simulink model "ofdm_txrx_mimo"  (Simulink 7.1)
settings: Solver=ode45  StartTime=0.0  StopTime=6e3  RelTol=1e-3
PreLoadFcn:
  | ofdm_rx_mimo_init
InitFcn:
  | ofdm_rx_mimo_init
StartFcn:
  | ofdm_rx_mimo_init
values from neighbouring files (symbols this model uses):
  BER_RAM_init_size = 2048    [ofdm_tx_mimo_init.m]
  CPLength = 16    [ofdm_tx_mimo_init.m]
  CRCPolynomial = hex2dec('04c11db7')    [ofdm_tx_mimo_init.m]
  freqOffset_track_filtCoef_i = hex2dec('2500')/2^32    [ofdm_rx_mimo_init.m]
  freqOffset_track_filtCoef_p = hex2dec('d0000')/2^32    [ofdm_rx_mimo_init.m]
  hi = zeros(1,length(cci))    [ofdm_rx_mimo_init.m]
  hr = zeros(1,length(ccr))    [ofdm_rx_mimo_init.m]
  max_CP_length = 16    [ofdm_tx_mimo_init.m]
  max_num_baseRateSymbols = 31    [ofdm_tx_mimo_init.m]
  max_num_subcarriers = 64    [ofdm_tx_mimo_init.m]
  max_num_trainingSymbols = 15    [ofdm_tx_mimo_init.m]
  max_numBytes = 16384    [ofdm_tx_mimo_init.m]
  max_OFDM_symbols = 2047    [ofdm_tx_mimo_init.m]
  modConstellation_qam16 = .75*[1 3 -1 -3]./3    [ofdm_tx_mimo_init.m]
  modConstellation_qam256 = 0.9375*[3 1 5 7 11 9 13 15 -3 -1 -5 -7 -11 -9 -13 -15]./15    [ofdm_tx_mimo_init.m]
  modConstellation_qam64 = 0.875*[3 1 5 7 -3 -1 -5 -7]./7    [ofdm_tx_mimo_init.m]
  modConstellation_qpsk = [1 -1]./sqrt(2)    [ofdm_tx_mimo_init.m]
  numBaseRateSymbols = 2    [ofdm_tx_mimo_init.m]
  numSubcarriers = 64    [ofdm_tx_mimo_init.m]
  numTrainingSymbols = 2    [ofdm_tx_mimo_init.m]
  pilot1_indicies = 7 + ( (64-7) * 2^16)    (= 3735559)    [ofdm_tx_mimo_init.m]
  pilot2_indicies = 21 + ( (64-21) * 2^16)    (= 2818069)    [ofdm_tx_mimo_init.m]
  pilotValue_neg = hex2dec('8000') + (2^16 * 0)    [ofdm_tx_mimo_init.m]
  pilotValue_pos = hex2dec('7FFF') + (2^16 * 0)    [ofdm_tx_mimo_init.m]
  pkt_crossCorr_thresh = 0.7    [ofdm_rx_mimo_init.m]
  pkt_energy_thresh = 0    [ofdm_rx_mimo_init.m]
  pkt_numPayloadBytes = 1400+42-6    (= 1436)    [ofdm_tx_mimo_init.m]
  pktByteNums = numHeaderBytes +  				byteIndex_numPayloadBytes(1)*2^8 +  				byteIndex_numPayloadBytes(2)*2^16 +  				byteIndex_simpleDynModMasks * 2^24    [ofdm_rx_mimo_init.m]
  PktDet_Delay = 26 + Symbol_Timing_Offset*2^7    (= 1050)    [ofdm_rx_mimo_init.m]
  preamble = 6*[0 shortsyms_10 longsyms_2]    [ofdm_tx_mimo_init.m]
  preamble_I = real(preamble)    [ofdm_tx_mimo_init.m]
  preamble_Q = imag(preamble)    [ofdm_tx_mimo_init.m]
  RAM_init_size = max_numBytes    (= 16384)    [ofdm_tx_mimo_init.m]
  reg_Interrupt_PktBuf_Control = reg_InterruptControl +  	1 * 2^16 +  	0 * 2^24    (= 65656)    [ofdm_rx_mimo_init.m]
  rx_controlBits = 1 * 2  		 + 1 * 4  		 + 0 * 8  		 + 1 * 16 * rx_SISO_Mode  		 + 1 * 32  		 + 1 * 64  		 + 0 * 128  		 + 1 * 256  		 + 0 * 512  		 + 1 * 1024  		 + 0 * 2048  		 + 1 * 4096  		 + 1 * 8192  		 + 0 * 16384  		 + 1 * 65536  		 + 1 * 131072  		 + 0 * 262144  		 + 1 * 524288    [ofdm_rx_mimo_init.m]
  Rx_PhaseNoiseTrack_K = 0.6094    [ofdm_rx_mimo_init.m]
  rx_postEq_scaling = round(rxScaling*2^11) * (1 + 2^16)    [ofdm_rx_mimo_init.m]
  RxDataScrambling_Seq = [40 198 78 63 82 173 102 245 48 111 172 115 147 230 216 93 72 65 62 2 205 242 122 90 128 83 105 97 73 10 5 252]    [ofdm_tx_mimo_init.m]
  simOnly_numSamples = length(preamble)+( (numSubcarriers+CPLength)*(numTrainingSymbols + numBaseRateSymbols + numFullRateSymbols/2) )    [ofdm_tx_mimo_init.m]
  train = [train train]    [ofdm_tx_mimo_init.m]
  tx_controlBits = (antB_preambleShift * 2^4) + (2^0 * tx_SISO_Mode) + 4*0 + 8*1    (= 73)    [ofdm_tx_mimo_init.m]
  TxDataScrambling_Seq = [40 198 78 63 82 173 102 245 48 111 172 115 147 230 216 93 72 65 62 2 205 242 122 90 128 83 105 97 73 10 5 252]    [ofdm_tx_mimo_init.m]
  txReg_symbolCounts = (2^16 * numFullRateSymbols) + (2^8 *numBaseRateSymbols ) + numTrainingSymbols    [ofdm_tx_mimo_init.m]
  TxRx_FFTScaling = bin2dec('010111') + (bin2dec('000101') * 2^6)    [ofdm_tx_mimo_init.m]
undefined symbols (not defined in the file or in its folder): AntA, AntB, BaseRate, CRC_table_gen, gcbh, intfilt, pktTiming_count_payloadStart, pktTiming_symbol_length, xlBlockLoadCallback, xlGetSimulinkPeriod
libraries: xbsIndex_r4 x4212, dspmlti4 x4, dspsigops x4, commchan3 x2, simulink x2, commrflib2 x2, dspsrcs4 x1
(part 5 of 43)

== system ofdm_txrx_mimo/OFDM Rx MIMO/Long Corr 4x ==
  note: changed latency to zero
  note: changed latency to 0
  I In : Inport  OutDataType=sfix(16)  OutScaling=2^0
  Q In : Inport  Port=2  OutDataType=sfix(16)  OutScaling=2^0
  AddSub : xbsIndex_r4/AddSub  mode=Subtraction  use_carryin=off  use_carryout=off  en=off  latency=0  precision=Full  arith_type=Unsigned  n_bits=8  bin_pt=2  quantization=Truncate  overflow=Wrap  dbl_ovrd=off  use_behavioral_HDL=on  pipelined=off  use_rpm=off  hw_selection=Fabric  block_type=addsub
      settings: xl_area=[5 0 0 8 0 0 0]  block_version=VER_STRING_GOES_HERE
  AddSub1 : xbsIndex_r4/AddSub  mode=Addition  use_carryin=off  use_carryout=off  en=off  latency=0  precision=Full  arith_type=Unsigned  n_bits=8  bin_pt=2  quantization=Truncate  overflow=Wrap  dbl_ovrd=off  use_behavioral_HDL=on  pipelined=off  use_rpm=off  hw_selection=Fabric  block_type=addsub
      settings: xl_area=[5 0 0 8 0 0 0]  block_version=VER_STRING_GOES_HERE
  AddSub2 : xbsIndex_r4/AddSub  mode=Addition  use_carryin=off  use_carryout=off  en=off  latency=0  precision=Full  arith_type=Unsigned  n_bits=8  bin_pt=2  quantization=Truncate  overflow=Wrap  dbl_ovrd=off  use_behavioral_HDL=on  pipelined=off  use_rpm=off  hw_selection=Fabric  block_type=addsub
      settings: xl_area=[10 0 0 18 0 0 0]  block_version=VER_STRING_GOES_HERE
  C1 : Subsystem  AncestorBlock=ofdm_rx_pom36_cfglib/correlator2/Original Subsystem/C1  mask{ns=0, N=4, h=hr(1:65), Ts_In=4}
      mask prompts: ns "Num sub-correlator"; N "Coefficients/correlator"; h "Coefficients"; Ts_In "Input sample period"
  C4 : Subsystem  AncestorBlock=ofdm_rx_pom36_cfglib/correlator2/Original Subsystem/C1  mask{ns=0, N=4, h=hi(1:65), Ts_In=4}
      mask prompts: ns "Num sub-correlator"; N "Coefficients/correlator"; h "Coefficients"; Ts_In "Input sample period"
  C6 : Subsystem  AncestorBlock=ofdm_rx_pom36_cfglib/correlator2/Original Subsystem/C1  mask{ns=0, N=4, h=hi(1:65), Ts_In=4}
      mask prompts: ns "Num sub-correlator"; N "Coefficients/correlator"; h "Coefficients"; Ts_In "Input sample period"
  C7 : Subsystem  AncestorBlock=ofdm_rx_pom36_cfglib/correlator2/Original Subsystem/C1  mask{ns=0, N=4, h=hr(1:65), Ts_In=4}
      mask prompts: ns "Num sub-correlator"; N "Coefficients/correlator"; h "Coefficients"; Ts_In "Input sample period"
  Gateway Out{3..8} (6 alike) : xbsIndex_r4/Gateway Out  hdl_port=off  timing_constraint=None  locs_specified=off  LOCs={}  block_type=gatewayout
      settings: infoedit="Gateway out block.  Converts Xilinx fixed point inputs into ouputs of type Simulink integer, double, or fixed point.<P><P>Hardware notes:  In hardware these blocks become top level output ports or are discarded, depending on how they are configured."  xl_area=[0 0 0 0 0 0 0]  block_version=VER_STRING_GOES_HERE
  Long Corr Scope4x : Scope  NumInputPorts=6
      settings: YMin=-1~-1~-30~-30~-20~-25  YMax=1~1~70~70~20~20  SaveName=ScopeData2
  Mult : xbsIndex_r4/Mult  precision=Full  arith_type="Signed  (2's comp)"  n_bits=8  bin_pt=2  quantization=Truncate  overflow=Wrap  en=off  latency=1  dbl_ovrd=off  use_behavioral_HDL=off  use_embedded=on  opt=Speed  optimum_pipeline=off  pipeline=on  use_rpm=off  placement_style=Rectangular shape  block_type=mult
      settings: infoedit=Hardware notes: To use the internal pipeline stage of the dedicated multiplier you must select 'Pipeline for maximum performance'.  xl_area=[60 18 0 106 0 0 0]  block_version=VER_STRING_GOES_HERE
  Mult1 : xbsIndex_r4/Mult  precision=Full  arith_type="Signed  (2's comp)"  n_bits=8  bin_pt=2  quantization=Truncate  overflow=Wrap  en=off  latency=1  dbl_ovrd=off  use_behavioral_HDL=off  use_embedded=on  opt=Speed  optimum_pipeline=off  pipeline=on  use_rpm=off  placement_style=Rectangular shape  block_type=mult
      settings: infoedit=Hardware notes: To use the internal pipeline stage of the dedicated multiplier you must select 'Pipeline for maximum performance'.  xl_area=[60 18 0 106 0 0 0]  block_version=VER_STRING_GOES_HERE
  Threshold1 : xbsIndex_r4/Threshold  en=off  latency=0  dbl_ovrd=off  block_type=sgn
      settings: infoedit="Presents -1 as output if input is negative; otherwise presents 1.  Output is a two bit signed number."  xl_area=[0 0 0 0 0 0 0]  block_version=VER_STRING_GOES_HERE
  Threshold2 : xbsIndex_r4/Threshold  en=off  latency=0  dbl_ovrd=off  block_type=sgn
      settings: infoedit="Presents -1 as output if input is negative; otherwise presents 1.  Output is a two bit signed number."  xl_area=[0 0 0 0 0 0 0]  block_version=VER_STRING_GOES_HERE
  y : Outport  OutDataType=sfix(16)  OutScaling=2^0
  wires:
  Gateway Out8/1 -> Long Corr Scope4x/5
  Gateway Out7/1 -> Long Corr Scope4x/4
  Gateway Out6/1 -> Long Corr Scope4x/3
  Gateway Out5/1 -> Long Corr Scope4x/6
  Gateway Out4/1 -> Long Corr Scope4x/2
  Gateway Out3/1 -> Long Corr Scope4x/1
  Threshold1/1 -> C4/1(x), C7/1(x)
  Threshold2/1 -> C1/1(x), C6/1(x)
  I In/1 -> Gateway Out3/1, Threshold2/1
  Q In/1 -> Gateway Out4/1, Threshold1/1
  C4/1(y) -> Gateway Out7/1, AddSub/2
  C6/1(y) -> Gateway Out8/1, AddSub1/1
  C7/1(y) -> Gateway Out5/1, AddSub1/2
  AddSub/1 -> Mult/1, Mult/2
  AddSub1/1 -> Mult1/1, Mult1/2
  Mult/1 -> AddSub2/1
  Mult1/1 -> AddSub2/2
  AddSub2/1 -> y/1
  C1/1(y) -> Gateway Out6/1, AddSub/1

== system ofdm_txrx_mimo/OFDM Rx MIMO/Packet_Detection ==
  Pkt Active : Inport  OutDataType=sfix(16)  OutScaling=2^0
  Reset : Inport  Port=2  OutDataType=sfix(16)  OutScaling=2^0
  Payload : Inport  Port=3  OutDataType=sfix(16)  OutScaling=2^0
  Internal PktDet (Sim Only!) : Subsystem
  Logical5 : xbsIndex_r4/Logical  logical_function=OR  inputs=2  en=off  latency=0  precision=Full  arith_type=Unsigned  n_bits=16  bin_pt=0  align_bp=on  dbl_ovrd=off  block_type=logical
      settings: block_version=8.2
  RSSI-based PktDet : Subsystem
  Simulation Multiplexer : xbsIndex_r4/Simulation Multiplexer  sim_sel=1  hw_sel=2  block_type=simmux
      settings: infoedit="Distinguish input subsystems as \"simulation only\" and \"generation only\".  The input specified For Simulation will be used during Simulink simulation.  The input specified For Generation will be used during code generation.  This block will typically be used on the output of a HW Co-Simulation, Black Box, or ModelSim subsystem.<P><P>Hardware Notes: This block costs nothing."  block_version=10.1.3
  Pkt Det : Outport  OutDataType=sfix(16)  OutScaling=2^0
  wires:
  Internal PktDet (Sim Only!)/1(PktDet) -> Simulation Multiplexer/1
  [AntA_ADC_I_Filt] -> Internal PktDet (Sim Only!)/1(AntA I)
  [AntA_ADC_Q_Filt] -> Internal PktDet (Sim Only!)/2(AntA Q)
  Simulation Multiplexer/1 -> Pkt Det/1
  Reset/1 -> RSSI-based PktDet/1(PktDet Reset)
  Payload/1 -> RSSI-based PktDet/2(Payload)
  Logical5/1 -> RSSI-based PktDet/3(Force Busy)
  Pkt Active/1 -> Logical5/1
  [Tx_Running] -> Logical5/2
  RSSI-based PktDet/1(PktDet) -> Simulation Multiplexer/2

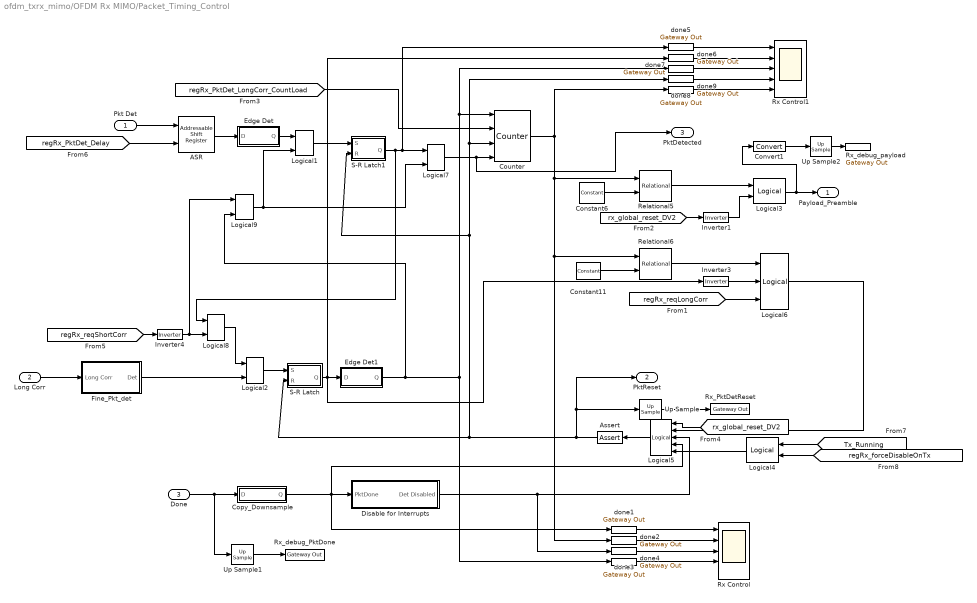
== system ofdm_txrx_mimo/OFDM Rx MIMO/Packet_Timing_Control ==
  Pkt Det : Inport  OutDataType=sfix(16)  OutScaling=2^0
  Long Corr : Inport  Port=2  OutDataType=sfix(16)  OutScaling=2^0
  Done : Inport  Port=3  OutDataType=sfix(16)  OutScaling=2^0
  ASR : xbsIndex_r4/Addressable Shift Register  infer_latency=on  depth=2  initVector=[0]  en=off  dbl_ovrd=off  use_behavioral_HDL=off  use_rpm=off  block_type=addrsr
      settings: infoedit="Delay of  configurable length.  Any element  in the delay     line   can    be   addressed   and   driven    onto   the   output     port.<P><P>Hardware  notes: Implemented  using SRL16s.     If Virtex-4, Virtex-II or Spartan-3 devices are used, multiple SRLC16s are cascaded together."  xl_area=[13 0 0 25 0 0 0]  block_version=VER_STRING_GOES_HERE
  Assert : xbsIndex_r4/Assert  Orientation=left  assert_type=on  type_source=Explicitly  arith_type=Boolean  n_bits=16  bin_pt=14  assert_rate=on  rate_source=Explicitly  period=4  output_port=on  block_type=assert
      settings: infoedit=Asserts a user-defined sample rate and/or type on a signal.<P><P>Hardware notes: In hardware this block costs nothing.  block_version=VER_STRING_GOES_HERE
  Constant11 : xbsIndex_r4/Constant  arith_type=Unsigned  const=pktTiming_count_payloadStart-2  n_bits=ceil(log2(pktTiming_count_payloadStart))  bin_pt=0  explicit_period=off  period=1  equ=P=C  opselect=C  inp2=PCIN>>17  opr=+  inp1=P  carry=CIN  dbl_ovrd=off  block_type=constant
      settings: block_version=VER_STRING_GOES_HERE
  Constant6 : xbsIndex_r4/Constant  arith_type=Unsigned  const=pktTiming_count_payloadStart  n_bits=ceil(log2(pktTiming_count_payloadStart))+1  bin_pt=0  explicit_period=off  period=1  equ=P=C  opselect=C  inp2=PCIN>>17  opr=+  inp1=P  carry=CIN  dbl_ovrd=off  block_type=constant
      settings: block_version=VER_STRING_GOES_HERE
  Convert1 : xbsIndex_r4/Convert  arith_type=Unsigned  n_bits=1  bin_pt=0  quantization=Truncate  overflow=Wrap  latency=0  dbl_ovrd=off  pipeline=off  block_type=convert
      settings: xl_area=[0, 0, 0, 0, 0, 0, 0]  block_version=VER_STRING_GOES_HERE
  Copy_Downsample : Subsystem
  Counter : xbsIndex_r4/Counter  cnt_type=Free Running  cnt_to=Inf  operation=Up  start_count=0  cnt_by_val=1  arith_type=Unsigned  n_bits=pktTiming_controlCounter_bits  bin_pt=0  load_pin=on  rst=on  en=on  explicit_period=off  period=1  dbl_ovrd=off  use_behavioral_HDL=off  use_rpm=on  implementation=Fabric  block_type=counter
      settings: xl_area=[6 11 0 12 0 0 0]  block_version=VER_STRING_GOES_HERE
  Disable for Interrupts : Subsystem
  Edge Det : Subsystem
  Edge Det1 : Subsystem
  Fine_Pkt_det : Subsystem
  Inverter1 : xbsIndex_r4/Inverter  en=off  latency=0  dbl_ovrd=off  block_type=inv
      settings: xl_area=[0 0 0 0 0 0 0]  block_version=VER_STRING_GOES_HERE
  Inverter3 : xbsIndex_r4/Inverter  en=off  latency=0  dbl_ovrd=off  block_type=inv
      settings: xl_area=[0 0 0 0 0 0 0]  block_version=VER_STRING_GOES_HERE
  Inverter4 : xbsIndex_r4/Inverter  en=off  latency=0  dbl_ovrd=off  block_type=inv
      settings: xl_area=[0 0 0 0 0 0 0]  block_version=VER_STRING_GOES_HERE
  Logical1 : xbsIndex_r4/Logical  logical_function=OR  inputs=2  en=off  latency=0  precision=Full  arith_type=Unsigned  n_bits=8  bin_pt=2  align_bp=on  dbl_ovrd=off  block_type=logical
      settings: xl_area=[1 0 0 1 0 0 0]  block_version=VER_STRING_GOES_HERE
  Logical2 : xbsIndex_r4/Logical  logical_function=AND  inputs=2  en=off  latency=0  precision=Full  arith_type=Unsigned  n_bits=8  bin_pt=2  align_bp=on  dbl_ovrd=off  block_type=logical
      settings: xl_area=[1 0 0 1 0 0 0]  block_version=VER_STRING_GOES_HERE
  Logical3 : xbsIndex_r4/Logical  logical_function=AND  inputs=2  en=off  latency=0  precision=Full  arith_type=Unsigned  n_bits=8  bin_pt=2  align_bp=on  dbl_ovrd=off  block_type=logical
      settings: xl_area=[1 0 0 1 0 0 0]  block_version=VER_STRING_GOES_HERE
  Logical4 : xbsIndex_r4/Logical  Orientation=left  logical_function=AND  inputs=2  en=off  latency=0  precision=Full  arith_type=Unsigned  n_bits=8  bin_pt=2  align_bp=on  dbl_ovrd=off  block_type=logical
      settings: xl_area=[1 0 0 1 0 0 0]  block_version=VER_STRING_GOES_HERE
  Logical5 : xbsIndex_r4/Logical  Orientation=left  logical_function=OR  inputs=5  en=off  latency=0  precision=Full  arith_type=Unsigned  n_bits=8  bin_pt=2  align_bp=on  dbl_ovrd=off  block_type=logical
      settings: xl_area=[1 0 0 1 0 0 0]  block_version=VER_STRING_GOES_HERE
  Logical6 : xbsIndex_r4/Logical  logical_function=AND  inputs=3  en=off  latency=0  precision=Full  arith_type=Unsigned  n_bits=8  bin_pt=2  align_bp=on  dbl_ovrd=off  block_type=logical
      settings: xl_area=[1 0 0 1 0 0 0]  block_version=VER_STRING_GOES_HERE
  Logical7 : xbsIndex_r4/Logical  logical_function=OR  inputs=2  en=off  latency=0  precision=Full  arith_type=Unsigned  n_bits=8  bin_pt=2  align_bp=on  dbl_ovrd=off  block_type=logical
      settings: xl_area=[1 0 0 1 0 0 0]  block_version=VER_STRING_GOES_HERE
  Logical8 : xbsIndex_r4/Logical  logical_function=OR  inputs=2  en=off  latency=0  precision=Full  arith_type=Unsigned  n_bits=8  bin_pt=2  align_bp=on  dbl_ovrd=off  block_type=logical
      settings: xl_area=[1 0 0 1 0 0 0]  block_version=VER_STRING_GOES_HERE
  Logical9 : xbsIndex_r4/Logical  logical_function=AND  inputs=2  en=off  latency=0  precision=Full  arith_type=Unsigned  n_bits=8  bin_pt=2  align_bp=on  dbl_ovrd=off  block_type=logical
      settings: xl_area=[1 0 0 1 0 0 0]  block_version=VER_STRING_GOES_HERE
  Relational5 : xbsIndex_r4/Relational  mode=a>b  en=off  latency=0  dbl_ovrd=off  block_type=relational
      settings: xl_area=[6 0 0 11 0 0 0]  block_version=VER_STRING_GOES_HERE
  Relational6 : xbsIndex_r4/Relational  mode=a>b  en=off  latency=0  dbl_ovrd=off  block_type=relational
      settings: xl_area=[6 0 0 11 0 0 0]  block_version=VER_STRING_GOES_HERE
  Rx Control : Scope  NumInputPorts=5
      settings: TimeRange=2500  YMin=0.95~885~-1~-1~-1  YMax=1.05~935~1~1~1  SaveName=ScopeData13
  Rx Control1 : Scope  NumInputPorts=5
      settings: TimeRange=2500  YMin=0.95~0.95~-1~-1~605  YMax=1.05~1.05~1~1~665  SaveName=ScopeData47
  Rx_PktDetReset : xbsIndex_r4/Gateway Out  hdl_port=on  timing_constraint=None  locs_specified=off  LOCs={}  block_type=gatewayout
      settings: infoedit="Gateway out block.  Converts Xilinx fixed point inputs into ouputs of type Simulink integer, double, or fixed point.<P><P>Hardware notes:  In hardware these blocks become top level output ports or are discarded, depending on how they are configured."  xl_area=[0, 0, 0, 0, 0, 0, 0]  block_version=VER_STRING_GOES_HERE
  Rx_debug_PktDone : xbsIndex_r4/Gateway Out  hdl_port=on  timing_constraint=None  locs_specified=off  LOCs={}  block_type=gatewayout
      settings: infoedit="Gateway out block.  Converts Xilinx fixed point inputs into ouputs of type Simulink integer, double, or fixed point.<P><P>Hardware notes:  In hardware these blocks become top level output ports or are discarded, depending on how they are configured."  xl_area=[0, 0, 0, 0, 0, 0, 0]  block_version=VER_STRING_GOES_HERE
  Rx_debug_payload : xbsIndex_r4/Gateway Out  hdl_port=on  timing_constraint=None  locs_specified=off  LOCs={}  block_type=gatewayout
      settings: infoedit="Gateway out block.  Converts Xilinx fixed point inputs into ouputs of type Simulink integer, double, or fixed point.<P><P>Hardware notes:  In hardware these blocks become top level output ports or are discarded, depending on how they are configured."  xl_area=[0 0 0 0 1 0 0]  block_version=VER_STRING_GOES_HERE
  S-R Latch : Subsystem
  S-R Latch1 : Subsystem
  Up Sample : xbsIndex_r4/Up Sample  sample_ratio=4  copy_samples=on  dbl_ovrd=off  block_type=usamp
      settings: infoedit="Up samples input data.  Inserted values  can be zeros or copies of the most recent input sample.<P><P>Hardware notes: No hardware is needed if inserted values are copies of the input sample; otherwise, a mux and single bit flip-flop are used."  block_version=8.2.02
  Up Sample1 : xbsIndex_r4/Up Sample  sample_ratio=2  copy_samples=on  dbl_ovrd=off  block_type=usamp
      settings: infoedit="Up samples input data.  Inserted values  can be zeros or copies of the most recent input sample.<P><P>Hardware notes: No hardware is needed if inserted values are copies of the input sample; otherwise, a mux and single bit flip-flop are used."  block_version=8.2.02
  Up Sample2 : xbsIndex_r4/Up Sample  sample_ratio=4  copy_samples=on  dbl_ovrd=off  block_type=usamp
      settings: infoedit="Up samples input data.  Inserted values  can be zeros or copies of the most recent input sample.<P><P>Hardware notes: No hardware is needed if inserted values are copies of the input sample; otherwise, a mux and single bit flip-flop are used."  block_version=8.2.02
  done{1..9} (9 alike) : xbsIndex_r4/Gateway Out  hdl_port=off  timing_constraint=None  locs_specified=off  LOCs={}  block_type=gatewayout
      settings: infoedit="Gateway out block.  Converts Xilinx fixed point inputs into ouputs of type Simulink integer, double, or fixed point.<P><P>Hardware notes:  In hardware these blocks become top level output ports or are discarded, depending on how they are configured."  xl_area=[0 0 0 0 1 0 0]  block_version=VER_STRING_GOES_HERE
  Payload_Preamble : Outport  OutDataType=sfix(16)  OutScaling=2^0
  PktReset : Outport  Port=2  OutDataType=sfix(16)  OutScaling=2^0
  PktDetected : Outport  Port=3  OutDataType=sfix(16)  OutScaling=2^0
  wires:
  Convert1/1 -> Up Sample2/1
  done9/1 -> Rx Control1/5
  done8/1 -> Rx Control1/4
  done7/1 -> Rx Control1/3
  done6/1 -> Rx Control1/2
  done5/1 -> Rx Control1/1
  Copy_Downsample/1(Q) -> done1/1, Logical5/4, Disable for Interrupts/1(PktDone)
  Assert/1 -> S-R Latch1/2(R), done8/1, Counter/3, S-R Latch/2(R), PktReset/1, Up Sample/1
  Disable for Interrupts/1(Det Disabled) -> done3/1, Logical5/3
  Done/1 -> Copy_Downsample/1(D), Up Sample1/1
  S-R Latch1/1(Q) -> done5/1, Logical7/1, Logical8/1
  Logical1/1 -> S-R Latch1/1(S)
  S-R Latch/1(Q) -> done6/1, Inverter3/1, Edge Det1/1(D)
  Edge Det1/1(Q) -> done7/1, Counter/1, done4/1, Logical9/2
  Logical9/1 -> Logical7/2, Logical1/2
  [regRx_PktDet_LongCorr_CountLoad] -> Counter/2
  Logical8/1 -> Logical2/1
  Inverter4/1 -> Logical9/1, Logical8/2
  [regRx_reqShortCorr] -> Inverter4/1
  Logical2/1 -> S-R Latch/1(S)
  Long Corr/1 -> Fine_Pkt_det/1(Long Corr)
  done4/1 -> Rx Control/4
  done3/1 -> Rx Control/3
  done2/1 -> Rx Control/2
  done1/1 -> Rx Control/1
  Logical7/1 -> Counter/4, PktDetected/1
  Logical6/1 -> Logical5/2
  [regRx_reqLongCorr] -> Logical6/3
  Inverter3/1 -> Logical6/2
  Relational6/1 -> Logical6/1
  Constant11/1 -> Relational6/2
  [rx_global_reset_DV2] -> Logical5/1
  Logical5/1 -> Assert/1
  Logical3/1 -> Convert1/1, Payload_Preamble/1
  Relational5/1 -> Logical3/1
  Inverter1/1 -> Logical3/2
  [rx_global_reset_DV2] -> Inverter1/1
  [regRx_PktDet_Delay] -> ASR/2
  ASR/1 -> Edge Det/1(D)
  Pkt Det/1 -> ASR/1
  Constant6/1 -> Relational5/2
  Edge Det/1(Q) -> Logical1/1
  Counter/1 -> done2/1, Relational6/1, Relational5/1, done9/1
  Fine_Pkt_det/1(Det) -> Logical2/2
  Up Sample/1 -> Rx_PktDetReset/1
  Up Sample1/1 -> Rx_debug_PktDone/1
  Up Sample2/1 -> Rx_debug_payload/1
  Logical4/1 -> Logical5/5
  [Tx_Running] -> Logical4/1
  [regRx_forceDisableOnTx] -> Logical4/2

== system ofdm_txrx_mimo/OFDM Rx MIMO/Rx Reset Logic ==
  Convert : xbsIndex_r4/Convert  arith_type=Boolean  n_bits=8  bin_pt=6  quantization=Truncate  overflow=Wrap  latency=0  dbl_ovrd=off  pipeline=off  block_type=convert
      settings: xl_area=[0, 0, 0, 0, 0, 0, 0]  block_version=VER_STRING_GOES_HERE
  Down Sample6 : xbsIndex_r4/Down Sample  sample_ratio=2  sample_phase="Last Value of Frame  (most efficient)"  en=off  latency=1  dbl_ovrd=off  block_type=dsamp
      settings: block_version=VER_STRING_GOES_HERE
  Logical1 : xbsIndex_r4/Logical  logical_function=OR  inputs=2  en=off  latency=0  precision=Full  arith_type=Unsigned  n_bits=8  bin_pt=2  align_bp=on  dbl_ovrd=off  block_type=logical
      settings: xl_area=[1 0 0 1 0 0 0]  block_version=VER_STRING_GOES_HERE
  RXreset : Constant  Value=0  OutDataType=sfix(16)  OutScaling=2^0
  Rx_Reset : xbsIndex_r4/Gateway In  arith_type=Boolean  n_bits=6  bin_pt=0  quantization="Round  (unbiased: +/- Inf)"  overflow=Saturate  period=4  dbl_ovrd=off  timing_constraint=None  locs_specified=off  LOCs={}  block_type=gatewayin
      settings: infoedit="Gateway in block.  Converts inputs of type Simulink integer, double and fixed point to  Xilinx fixed point type.<P><P>Hardware notes:  In hardware these blocks become top level input ports."  xl_area=[0 0 0 0 1 0 0]  block_version=VER_STRING_GOES_HERE
  tag scope: rx_global_reset=global, rx_global_reset_DV2=global
  wires:
  RXreset/1 -> Rx_Reset/1
  [regRx_GlobalReset] -> Logical1/1
  Logical1/1 -> [rx_global_reset], Down Sample6/1
  Rx_Reset/1 -> Convert/1
  Convert/1 -> Logical1/2
  Down Sample6/1 -> [rx_global_reset_DV2]

== system ofdm_txrx_mimo/OFDM Tx MIMO/Filter & DACs ==
  A_I : Inport  OutDataType=sfix(16)  OutScaling=2^0
  A_Q : Inport  Port=2  OutDataType=sfix(16)  OutScaling=2^0
  B_I : Inport  Port=3  OutDataType=sfix(16)  OutScaling=2^0
  B_Q : Inport  Port=4  OutDataType=sfix(16)  OutScaling=2^0
  Convert : xbsIndex_r4/Convert  arith_type=Boolean  n_bits=16  bin_pt=14  quantization=Truncate  overflow=Wrap  latency=0  dbl_ovrd=off  pipeline=off  block_type=convert
      settings: block_version=8.2.02
  Down Sample : xbsIndex_r4/Down Sample  sample_ratio=2  sample_phase="Last Value of Frame  (most efficient)"  en=off  latency=1  dbl_ovrd=off  block_type=dsamp
      settings: block_version=10.1
  Logical : xbsIndex_r4/Logical  logical_function=AND  inputs=2  en=off  latency=0  precision=Full  arith_type=Unsigned  n_bits=16  bin_pt=0  align_bp=on  dbl_ovrd=off  block_type=logical
      settings: block_version=8.2.02
  Mux : xbsIndex_r4/Mux  inputs=2  en=off  latency=1  precision=Full  arith_type=Unsigned  n_bits=16  bin_pt=14  quantization=Truncate  overflow=Wrap  dbl_ovrd=off  block_type=mux
      settings: block_version=8.2.02
  Mux1 : xbsIndex_r4/Mux  inputs=2  en=off  latency=1  precision=Full  arith_type=Unsigned  n_bits=16  bin_pt=14  quantization=Truncate  overflow=Wrap  dbl_ovrd=off  block_type=mux
      settings: block_version=8.2.02
  Polyphase Interpolator : Subsystem  mask{h=[0 intfilt(4,4,0.8)];, polyphaseBranches=4, data_samplePeriod=4, coeff_prec=14, coeff_bp=13, multLatency=1, mult_prec=18, mult_bp=17, add_prec=16, add_bp=15}
      mask prompts: h "Filter Coefficients"; polyphaseBranches "Interpolation Rate"; data_samplePeriod "Input Sample Period"; coeff_prec "Coefficients - Num Bits"; coeff_bp "Coefficients - Binary Point"; multLatency "Multiplier Latency"; mult_prec "Multiplier - Num Bits"; mult_bp "Multiplier - Binary Point"; add_prec "Final Adder - Num Bits"; add_bp "Final Adder - Binary Point"
      mask help:
      | This block implements a interpolating polyphase FIR filter. In this version,
      | the interpolation rate must be 8, and the filter must be of length 64.
      mask init:
      | subFilterLength = 4;
  Polyphase Interpolator1 : Subsystem  mask{h=[0 intfilt(4,4,0.8)];, polyphaseBranches=4, data_samplePeriod=4, coeff_prec=14, coeff_bp=13, multLatency=1, mult_prec=18, mult_bp=17, add_prec=16, add_bp=15}
      mask prompts: h "Filter Coefficients"; polyphaseBranches "Interpolation Rate"; data_samplePeriod "Input Sample Period"; coeff_prec "Coefficients - Num Bits"; coeff_bp "Coefficients - Binary Point"; multLatency "Multiplier Latency"; mult_prec "Multiplier - Num Bits"; mult_bp "Multiplier - Binary Point"; add_prec "Final Adder - Num Bits"; add_bp "Final Adder - Binary Point"
      mask help:
      | This block implements a interpolating polyphase FIR filter. In this version,
      | the interpolation rate must be 8, and the filter must be of length 64.
      mask init:
      | subFilterLength = 4;
  Polyphase Interpolator2 : Subsystem  mask{h=[0 intfilt(4,4,0.8)];, polyphaseBranches=4, data_samplePeriod=4, coeff_prec=14, coeff_bp=13, multLatency=1, mult_prec=18, mult_bp=17, add_prec=16, add_bp=15}
      mask prompts: h "Filter Coefficients"; polyphaseBranches "Interpolation Rate"; data_samplePeriod "Input Sample Period"; coeff_prec "Coefficients - Num Bits"; coeff_bp "Coefficients - Binary Point"; multLatency "Multiplier Latency"; mult_prec "Multiplier - Num Bits"; mult_bp "Multiplier - Binary Point"; add_prec "Final Adder - Num Bits"; add_bp "Final Adder - Binary Point"
      mask help:
      | This block implements a interpolating polyphase FIR filter. In this version,
      | the interpolation rate must be 8, and the filter must be of length 64.
      mask init:
      | subFilterLength = 4;
  Polyphase Interpolator3 : Subsystem  mask{h=[0 intfilt(4,4,0.8)];, polyphaseBranches=4, data_samplePeriod=4, coeff_prec=14, coeff_bp=13, multLatency=1, mult_prec=18, mult_bp=17, add_prec=16, add_bp=15}
      mask prompts: h "Filter Coefficients"; polyphaseBranches "Interpolation Rate"; data_samplePeriod "Input Sample Period"; coeff_prec "Coefficients - Num Bits"; coeff_bp "Coefficients - Binary Point"; multLatency "Multiplier Latency"; mult_prec "Multiplier - Num Bits"; mult_bp "Multiplier - Binary Point"; add_prec "Final Adder - Num Bits"; add_bp "Final Adder - Binary Point"
      mask help:
      | This block implements a interpolating polyphase FIR filter. In this version,
      | the interpolation rate must be 8, and the filter must be of length 64.
      mask init:
      | subFilterLength = 4;
  Register : xbsIndex_r4/Register  init=0  rst=off  en=off  dbl_ovrd=off  block_type=register
      settings: block_version=8.2.02
  Register2 : xbsIndex_r4/Register  init=0  rst=off  en=off  dbl_ovrd=off  block_type=register
      settings: block_version=8.2.02
  Tx Scope : Scope  NumInputPorts=4
      settings: TimeRange=3000  YMin=-1~-1~-1~0.2375  YMax=1~1~1~0.2625  SaveName=ScopeData76
  Tx_AntA_DAC_I : xbsIndex_r4/Gateway Out  hdl_port=on  timing_constraint=None  locs_specified=off  LOCs={}  block_type=gatewayout
      settings: infoedit="Gateway out block.  Converts Xilinx fixed point inputs into ouputs of type Simulink integer, double, or fixed point.<P><P>Hardware notes:  In hardware these blocks become top level output ports or are discarded, depending on how they are configured."  block_version=10.1.3
  Tx_AntA_DAC_Q : xbsIndex_r4/Gateway Out  hdl_port=on  timing_constraint=None  locs_specified=off  LOCs={}  block_type=gatewayout
      settings: infoedit="Gateway out block.  Converts Xilinx fixed point inputs into ouputs of type Simulink integer, double, or fixed point.<P><P>Hardware notes:  In hardware these blocks become top level output ports or are discarded, depending on how they are configured."  block_version=10.1.3
  Tx_AntA_DAC_Q{1..4} (4 alike) : xbsIndex_r4/Gateway Out  hdl_port=off  timing_constraint=None  locs_specified=off  LOCs={}  block_type=gatewayout
      settings: infoedit="Gateway out block.  Converts Xilinx fixed point inputs into ouputs of type Simulink integer, double, or fixed point.<P><P>Hardware notes:  In hardware these blocks become top level output ports or are discarded, depending on how they are configured."  block_version=10.1.3
  Tx_AntB_DAC_I : xbsIndex_r4/Gateway Out  hdl_port=on  timing_constraint=None  locs_specified=off  LOCs={}  block_type=gatewayout
      settings: infoedit="Gateway out block.  Converts Xilinx fixed point inputs into ouputs of type Simulink integer, double, or fixed point.<P><P>Hardware notes:  In hardware these blocks become top level output ports or are discarded, depending on how they are configured."  block_version=10.1.3
  Tx_AntB_DAC_Q : xbsIndex_r4/Gateway Out  hdl_port=on  timing_constraint=None  locs_specified=off  LOCs={}  block_type=gatewayout
      settings: infoedit="Gateway out block.  Converts Xilinx fixed point inputs into ouputs of type Simulink integer, double, or fixed point.<P><P>Hardware notes:  In hardware these blocks become top level output ports or are discarded, depending on how they are configured."  block_version=10.1.3
  " A_I" : Outport  OutDataType=sfix(16)  OutScaling=2^0
  " A_Q" : Outport  Port=2  OutDataType=sfix(16)  OutScaling=2^0
  " B_I" : Outport  Port=3  OutDataType=sfix(16)  OutScaling=2^0
  " B_Q" : Outport  Port=4  OutDataType=sfix(16)  OutScaling=2^0
  wires:
  Polyphase Interpolator/1(Y) -> Mux/3, Register/1, Tx_AntA_DAC_Q2/1
  Tx_AntA_DAC_I/1 -> " A_I"/1
  Polyphase Interpolator1/1(Y) -> Mux1/3, Register2/1, Tx_AntA_DAC_Q4/1
  Tx_AntA_DAC_Q/1 -> " A_Q"/1
  Polyphase Interpolator2/1(Y) -> Mux/2
  Tx_AntB_DAC_I/1 -> " B_I"/1
  Polyphase Interpolator3/1(Y) -> Mux1/2
  Tx_AntB_DAC_Q/1 -> " B_Q"/1
  Mux/1 -> Tx_AntB_DAC_I/1
  Mux1/1 -> Tx_AntB_DAC_Q/1
  Logical/1 -> Down Sample/1
  Register/1 -> Tx_AntA_DAC_I/1
  Register2/1 -> Tx_AntA_DAC_Q/1
  [TxReg_SISO_Mode] -> Logical/1
  [TxReg_SISO_AntB] -> Convert/1
  Convert/1 -> Logical/2
  Down Sample/1 -> Mux/1, Mux1/1
  A_I/1 -> Polyphase Interpolator/1(X), Tx_AntA_DAC_Q1/1
  A_Q/1 -> Polyphase Interpolator1/1(X), Tx_AntA_DAC_Q3/1
  B_I/1 -> Polyphase Interpolator2/1(X)
  B_Q/1 -> Polyphase Interpolator3/1(X)
  Tx_AntA_DAC_Q1/1 -> Tx Scope/1
  Tx_AntA_DAC_Q2/1 -> Tx Scope/2
  Tx_AntA_DAC_Q3/1 -> Tx Scope/3
  Tx_AntA_DAC_Q4/1 -> Tx Scope/4

== system ofdm_txrx_mimo/OFDM Tx MIMO/IFFT ==
  xn_I : Inport  OutDataType=sfix(16)  OutScaling=2^0
  xn_Q : Inport  Port=2  OutDataType=sfix(16)  OutScaling=2^0
  Start : Inport  Port=3  OutDataType=sfix(16)  OutScaling=2^0
  Constant3 : xbsIndex_r4/Constant  arith_type=Boolean  const=0  n_bits=1  bin_pt=0  explicit_period=off  period=1  equ=P=C  opselect=C  inp2=PCIN>>17  opr=+  inp1=P  carry=CIN  dbl_ovrd=off  block_type=constant
      settings: block_version=VER_STRING_GOES_HERE
  Constant4 : xbsIndex_r4/Constant  arith_type=Boolean  const=1  n_bits=1  bin_pt=0  explicit_period=off  period=1  equ=P=C  opselect=C  inp2=PCIN>>17  opr=+  inp1=P  carry=CIN  dbl_ovrd=off  block_type=constant
      settings: block_version=VER_STRING_GOES_HERE
  Constant6 : xbsIndex_r4/Constant  arith_type=Boolean  const=1  n_bits=1  bin_pt=0  explicit_period=off  period=1  equ=P=C  opselect=C  inp2=PCIN>>17  opr=+  inp1=P  carry=CIN  dbl_ovrd=off  block_type=constant
      settings: block_version=VER_STRING_GOES_HERE
  FFTx : xbsIndex_r4/FFT v3_1   arch=Pipelined Streaming I/O  npts=64  scaling=Scaled  rounding=Convergent Rounding  tf_width=16  en_n=off  has_ovflo=on  rst=on  en=off  op_order=Natural Order  dbl_ovrd=off  explicit_period=off  period=4  use_dsp48=off  twiddle_mem_type=Block RAM  data_mem_type=Block RAM  num_bram_stages=3  block_type=fftx
      settings: infoedit=Devices Supported: Virtex-4, Virtex-II, Virtex-IIPro, Spartan-3 and Spartan-3E  xl_area=[0, 0, 0, 0, 0, 0, 0]  block_version=VER_STRING_GOES_HERE
  IFFT Input : Scope  NumInputPorts=4
      settings: TimeRange=4000  YMin=-0.8~-0.8~-1~0  YMax=1~0.8~1~65  SaveName=ScopeData16
  IFFT Output : Scope  NumInputPorts=6
      settings: TimeRange=4000  YMin=-0.6~-0.6~0~0~0~-1  YMax=0.2~0.2~70~1~1~1  SaveName=ScopeData17
  IFFT Output1 : Scope  NumInputPorts=6
      settings: TimeRange=4000  YMin=-0.6~-0.6~0~0~0~-1  YMax=0.2~0.2~70~1~1~1  SaveName=ScopeData69
  done{1..10} (10 alike) : xbsIndex_r4/Gateway Out  hdl_port=off  timing_constraint=None  locs_specified=off  LOCs={}  block_type=gatewayout
      settings: infoedit="Gateway out block.  Converts Xilinx fixed point inputs into ouputs of type Simulink integer, double, or fixed point.<P><P>Hardware notes:  In hardware these blocks become top level output ports or are discarded, depending on how they are configured."  xl_area=[0 0 0 0 1 0 0]  block_version=VER_STRING_GOES_HERE
  xn_Index : Outport  OutDataType=sfix(16)  OutScaling=2^0
  Vout : Outport  Port=2  OutDataType=sfix(16)  OutScaling=2^0
  xk_I : Outport  Port=3  OutDataType=sfix(16)  OutScaling=2^0
  xk_Q : Outport  Port=4  OutDataType=sfix(16)  OutScaling=2^0
  xk_Index : Outport  Port=5  OutDataType=sfix(16)  OutScaling=2^0
  wires:
  Constant3/1 -> FFTx/4
  Constant4/1 -> FFTx/5
  Constant6/1 -> FFTx/7
  [TxReset] -> FFTx/8
  xn_I/1 -> FFTx/1, done4/1
  FFTx/1 -> xk_I/1, done8/1
  FFTx/2 -> xk_Q/1, done9/1
  xn_Q/1 -> FFTx/2, done5/1
  FFTx/3 -> xn_Index/1, done6/1, IFFT Output1/2
  Start/1 -> FFTx/3, done3/1, IFFT Output1/1
  FFTx/4 -> xk_Index/1, done7/1, IFFT Output1/3
  FFTx/7 -> Vout/1, done10/1, IFFT Output1/4
  done4/1 -> IFFT Input/1
  done5/1 -> IFFT Input/2
  done3/1 -> IFFT Input/3
  done6/1 -> IFFT Input/4
  done8/1 -> IFFT Output/1
  done9/1 -> IFFT Output/2
  done7/1 -> IFFT Output/3
  done10/1 -> IFFT Output/4
  done2/1 -> IFFT Output/5
  FFTx/9 -> done2/1, IFFT Output1/5
  done1/1 -> IFFT Output/6
  FFTx/10 -> done1/1
  [Tx_FFTScaling] -> FFTx/6

== system ofdm_txrx_mimo/OFDM Tx MIMO/OutputBuffers ==
  IFFT Vout : Inport  OutDataType=sfix(16)  OutScaling=2^0
  I : Inport  Port=2  OutDataType=sfix(16)  OutScaling=2^0
  Q : Inport  Port=3  OutDataType=sfix(16)  OutScaling=2^0
  Xk_index : Inport  Port=4  OutDataType=sfix(16)  OutScaling=2^0
  1 Shift Reg : Subsystem
  Addr Gen : Subsystem
  Constant2 : xbsIndex_r4/Constant  arith_type=Boolean  const=0  n_bits=0  bin_pt=0  explicit_period=off  period=1  equ=P=C  opselect=C  inp2=PCIN>>17  opr=+  inp1=P  carry=CIN  dbl_ovrd=off  block_type=constant
      settings: block_version=VER_STRING_GOES_HERE
  Convert1 : xbsIndex_r4/Convert  arith_type="Signed  (2's comp)"  n_bits=14  bin_pt=13  quantization=Truncate  overflow=Flag as error  latency=0  dbl_ovrd=off  pipeline=off  block_type=convert
      settings: xl_area=[0 0 0 0 0 0 0]  block_version=VER_STRING_GOES_HERE
  Convert2 : xbsIndex_r4/Convert  arith_type="Signed  (2's comp)"  n_bits=14  bin_pt=13  quantization=Truncate  overflow=Flag as error  latency=0  dbl_ovrd=off  pipeline=off  block_type=convert
      settings: xl_area=[0 0 0 0 0 0 0]  block_version=VER_STRING_GOES_HERE
  Delay : xbsIndex_r4/Delay  en=off  latency=1  dbl_ovrd=off  reg_retiming=off  block_type=delay
      settings: infoedit=Hardware notes: A delay line is a chain, each link of which is an SRL16 followed by a flip-flop. If register retiming is enabled, the delay line is a chain of flip-flops.  block_version=VER_STRING_GOES_HERE
  Logical : xbsIndex_r4/Logical  logical_function=AND  inputs=2  en=off  latency=0  precision=Full  arith_type=Unsigned  n_bits=16  bin_pt=0  align_bp=on  dbl_ovrd=off  block_type=logical
      settings: block_version=VER_STRING_GOES_HERE
  Logical1 : xbsIndex_r4/Logical  logical_function=AND  inputs=2  en=off  latency=0  precision=Full  arith_type=Unsigned  n_bits=16  bin_pt=0  align_bp=on  dbl_ovrd=off  block_type=logical
      settings: block_version=VER_STRING_GOES_HERE
  Logical2 : xbsIndex_r4/Logical  logical_function=OR  inputs=2  en=off  latency=0  precision=Full  arith_type=Unsigned  n_bits=16  bin_pt=0  align_bp=on  dbl_ovrd=off  block_type=logical
      settings: block_version=VER_STRING_GOES_HERE
  Logical3 : xbsIndex_r4/Logical  logical_function=AND  inputs=2  en=off  latency=0  precision=Full  arith_type=Unsigned  n_bits=16  bin_pt=0  align_bp=on  dbl_ovrd=off  block_type=logical
      settings: block_version=VER_STRING_GOES_HERE
  Logical4 : xbsIndex_r4/Logical  logical_function=OR  inputs=2  en=off  latency=0  precision=Full  arith_type=Unsigned  n_bits=16  bin_pt=0  align_bp=on  dbl_ovrd=off  block_type=logical
      settings: block_version=VER_STRING_GOES_HERE
  Logical5 : xbsIndex_r4/Logical  logical_function=OR  inputs=2  en=off  latency=0  precision=Full  arith_type=Unsigned  n_bits=16  bin_pt=0  align_bp=on  dbl_ovrd=off  block_type=logical
      settings: block_version=VER_STRING_GOES_HERE
  Mem Control : Subsystem
  Output Buffer Scope : Scope  NumInputPorts=7
      settings: TimeRange=3000  YMin=0~0~0~0~0~0~0  YMax=1~1~1~1~150~150~1  SaveName=ScopeData5
  Pulse Generator1 : DiscretePulseGenerator  Period=simOnly_numSamples*2 + 500  PhaseDelay=(simOnly_numSamples*2 + 500) - 300
  RAMs : Subsystem
  Symbol Counter : xbsIndex_r4/Counter  cnt_type=Free Running  cnt_to=Inf  operation=Up  start_count=0  cnt_by_val=1  arith_type="Signed  (2's comp)"  n_bits=ceil(log2(max_OFDM_symbols+1))  bin_pt=0  load_pin=off  rst=on  en=on  explicit_period=off  period=1  dbl_ovrd=off  use_behavioral_HDL=off  use_rpm=on  implementation=Fabric  block_type=counter
      settings: xl_area=[4 7 0 7 0 0 0]  block_version=VER_STRING_GOES_HERE
  done{1..7} (7 alike) : xbsIndex_r4/Gateway Out  hdl_port=off  timing_constraint=None  locs_specified=off  LOCs={}  block_type=gatewayout
      settings: infoedit="Gateway out block.  Converts Xilinx fixed point inputs into ouputs of type Simulink integer, double, or fixed point.<P><P>Hardware notes:  In hardware these blocks become top level output ports or are discarded, depending on how they are configured."  xl_area=[0 0 0 0 1 0 0]  block_version=VER_STRING_GOES_HERE
  negedge : Subsystem
  AntA_I : Outport  OutDataType=sfix(16)  OutScaling=2^0
  AntA_Q : Outport  Port=2  OutDataType=sfix(16)  OutScaling=2^0
  AntB_I : Outport  Port=3  OutDataType=sfix(16)  OutScaling=2^0
  AntB_Q : Outport  Port=4  OutDataType=sfix(16)  OutScaling=2^0
  Vout : Outport  Port=5  OutDataType=sfix(16)  OutScaling=2^0
  Pkt Done : Outport  Port=6  OutDataType=sfix(16)  OutScaling=2^0
  wires:
  Q/1 -> Convert1/1
  I/1 -> Convert2/1
  Convert2/1 -> RAMs/8(I), done4/1
  Convert1/1 -> RAMs/9(Q), done3/1
  Xk_index/1 -> Addr Gen/4(Xk Index)
  [LocalReset] -> Symbol Counter/1
  Logical/1 -> RAMs/1(A We), done6/1
  Logical1/1 -> RAMs/2(B We), done5/1
  RAMs/1(AntA_I) -> AntA_I/1
  RAMs/2(AntA_Q) -> AntA_Q/1
  RAMs/3(AntB_I) -> AntB_I/1
  RAMs/4(AntB_Q) -> AntB_Q/1
  Mem Control/2(weA) -> Logical/1
  Mem Control/3(weB) -> Logical1/2
  [LocalReset] -> Addr Gen/5(rst)
  Mem Control/5(BaseRateSym) -> Addr Gen/2(BaseRateSym)
  Symbol Counter/1 -> Mem Control/1(FFTsymNum)
  Addr Gen/2(OE#A) -> RAMs/3(A OE#)
  Addr Gen/3(OE#B) -> RAMs/4(B OE#)
  Addr Gen/4(Wr Addr) -> RAMs/5(Wr Addr), done2/1
  Addr Gen/5(Rd Addr) -> RAMs/6(Rd Addr), done1/1
  IFFT Vout/1 -> Logical/2, Logical1/1, negedge/1(D)
  negedge/1(Q) -> Symbol Counter/2, Addr Gen/3(SymWrDone)
  Mem Control/4(TrainingSym) -> Addr Gen/1(TrainingSym)
  Logical2/1 -> Logical4/1
  [TxReset] -> Logical2/2
  Addr Gen/1(LastSamp) -> Logical3/2, 1 Shift Reg/3(en)
  Logical3/1 -> Logical5/1, Delay/1
  Constant2/1 -> Logical2/1
  RAMs/5(Rd Vout) -> Vout/1
  Addr Gen/6(Rd Vout) -> RAMs/7(Rd Vin), done7/1
  Logical4/1 -> [LocalReset]
  Mem Control/1(LastSym) -> 1 Shift Reg/1(d)
  [LocalReset] -> 1 Shift Reg/2(rst)
  1 Shift Reg/1(Q) -> Logical3/1
  done7/1 -> Output Buffer Scope/7
  done1/1 -> Output Buffer Scope/6
  done2/1 -> Output Buffer Scope/5
  done3/1 -> Output Buffer Scope/4
  done4/1 -> Output Buffer Scope/3
  done5/1 -> Output Buffer Scope/2
  done6/1 -> Output Buffer Scope/1
  Delay/1 -> Logical5/2
  Logical5/1 -> Pkt Done/1, Logical4/2
